simulink model "Simulatore_COMAU_UBHAND_IV_manipolazione_interna"  (Simulink 7.6)
settings: Solver=ode45  StartTime=0.0  StopTime=inf  RelTol=1e-3
values from neighbouring files (symbols this model uses):
  a_f = zeros(4,5)    [KinematicData.m]
  alpha_f = zeros(4,5)    [KinematicData.m]
  d_f = zeros(4,5)    [KinematicData.m]
  dc4_1 = 660    [KinematicData.m]
  T6HAND = [1 0 0 0;0 1 0 0; 0 0 1 357.48+95; 0 0 0 1]    [KinematicData.m]
  teta_d = atan(647.07/130)    [KinematicData.m]
  teta_f = zeros(4,5)    [KinematicData.m]
undefined symbols (not defined in the file or in its folder): a_c, a_w, alpha_c, alpha_w, d_c, d_w, Dt_fingers, teta_c
libraries: simulink x281, dspmtrx3 x171, aerolibutil x163, aerolibconvert2 x64, simulink_extras x46, vrlib x26, aerolibtransform2 x7, dspnetwork x1
(part 15 of 15)

== system Simulatore_COMAU_UBHAND_IV_manipolazione_interna/Work Space Control/Inverse_kinematic/Inverse_kinematic_comau/R_0index/T_6_index_^-1/Direct_kinematic_index1/Subsystem3 ==
  q : Inport
  alpha : Inport  Port=2
  a : Inport  Port=3
  A_01 : aerolibutil/Create 3x3 Matrix
  Constant : Constant  Value=0
  Constant1 : Constant  Value=[0 0 0 1]  VectorParams1D=off
  Constant5 : Constant  Value=0
  Display : Display
  Gain : Gain  Gain=-1
  Gain1 : Gain  Gain=-1
  Matrix Concatenation : Concatenate  Mode=Multidimensional array  ConcatenateDimension=2
  Matrix Concatenation1 : Concatenate  Mode=Multidimensional array
  Product{,1..5} (6 alike) : Product  RndMeth=Zero
  Trigonometric Function{,1,6..8,11..12} (7 alike) : Trigonometry  Operator=cos
  Trigonometric Function{2..5,9..10,13} (7 alike) : Trigonometry
  Vector Concatenate : Concatenate  NumInputs=3  Mode=Multidimensional array
  Out1 : Outport
  wires:
  Trigonometric Function2/1 -> Product/1
  Trigonometric Function1/1 -> Product/2
  q/1 -> Trigonometric Function/1, Trigonometric Function2/1, Trigonometric Function3/1, Trigonometric Function5/1, Trigonometric Function6/1, Trigonometric Function8/1, Trigonometric Function12/1, Trigonometric Function13/1
  alpha/1 -> Trigonometric Function1/1, Trigonometric Function4/1, Trigonometric Function7/1, Trigonometric Function9/1, Trigonometric Function10/1, Trigonometric Function11/1
  Trigonometric Function3/1 -> Product1/1
  Trigonometric Function4/1 -> Product1/2
  Trigonometric Function6/1 -> Product2/1
  Trigonometric Function7/1 -> Product2/2
  Trigonometric Function8/1 -> Product3/1
  Trigonometric Function9/1 -> Product3/2
  Product3/1 -> Gain1/1
  Product/1 -> Gain/1
  Trigonometric Function/1 -> A_01/1
  Gain/1 -> A_01/2
  Product1/1 -> A_01/3
  Trigonometric Function5/1 -> A_01/4
  Product2/1 -> A_01/5
  Gain1/1 -> A_01/6
  Constant/1 -> A_01/7
  Trigonometric Function10/1 -> A_01/8
  Trigonometric Function11/1 -> A_01/9
  a/1 -> Product5/1, Product4/1
  Trigonometric Function12/1 -> Product4/2
  Trigonometric Function13/1 -> Product5/2
  Product4/1 -> Vector Concatenate/1
  Product5/1 -> Vector Concatenate/2
  Constant5/1 -> Vector Concatenate/3
  A_01/1 -> Matrix Concatenation/1
  Vector Concatenate/1 -> Matrix Concatenation/2
  Matrix Concatenation/1 -> Matrix Concatenation1/1
  Constant1/1 -> Matrix Concatenation1/2
  Matrix Concatenation1/1 -> Out1/1, Display/1

== system Simulatore_COMAU_UBHAND_IV_manipolazione_interna/Work Space Control/Inverse_kinematic/Inverse_kinematic_comau/R_0index/T_6_index_^-1/Direct_kinematic_wrist1/T_6_8 ==
  wrist_angles[1..2] : Inport
  T_6H : Inport  Port=2
  Add : Sum
  Add1 : Sum
  Constant : Constant  Value=[alpha_w(1) alpha_w(2)]
  Constant1 : Constant  Value=0
  Constant2 : Constant  Value=[a_w(1) a_w(2)]
  Constant3 : Constant  Value=0
  Degrees to Radians : simulink_extras/Transformations/Degrees to Radians
  Demux : Demux  Outputs=2
  Demux1 : Demux  Outputs=2
  Demux2 : Demux  Outputs=2
  Display3 : Display
  Matrix Multiply : Product  Multiplication=Matrix(*)
  Matrix Multiply1 : Product  Multiplication=Matrix(*)
  Matrix Multiply2 : Product  Multiplication=Matrix(*)
  Subsystem : Subsystem
  Subsystem1 : Subsystem
  wrist_reference_ : Subsystem
  T_68 : Outport
  wires:
  wrist_angles[1..2]/1 -> Display3/1, Degrees to Radians/1
  Degrees to Radians/1 -> Demux/1
  Demux1/2 -> Subsystem1/2(alpha)
  Demux1/1 -> Subsystem/2(alpha)
  Demux2/2 -> Subsystem1/3(a)
  Demux2/1 -> Subsystem/3(a)
  Constant2/1 -> Demux2/1
  Matrix Multiply/1 -> T_68/1
  Subsystem1/1(Out1) -> Matrix Multiply/2
  Constant/1 -> Demux1/1
  Subsystem/1(Out1) -> Matrix Multiply1/2
  Matrix Multiply1/1 -> Matrix Multiply/1
  Constant1/1 -> Add/2
  Demux/1 -> Add/1
  Add/1 -> Subsystem/1(q)
  Constant3/1 -> Add1/2
  Demux/2 -> Add1/1
  Add1/1 -> Subsystem1/1(q)
  T_6H/1 -> Matrix Multiply2/1
  wrist_reference_/1(T_HW) -> Matrix Multiply2/2
  Matrix Multiply2/1 -> Matrix Multiply1/1

== system Simulatore_COMAU_UBHAND_IV_manipolazione_interna/Work Space Control/Inverse_kinematic/Inverse_kinematic_comau/R_0index/T_6_object^-1/Direct_kinematic_index1/Subsystem ==
  q : Inport
  alpha : Inport  Port=2
  a : Inport  Port=3
  A_01 : aerolibutil/Create 3x3 Matrix
  Constant : Constant  Value=0
  Constant1 : Constant  Value=[0 0 0 1]  VectorParams1D=off
  Constant5 : Constant  Value=0
  Display : Display
  Gain : Gain  Gain=-1
  Gain1 : Gain  Gain=-1
  Matrix Concatenation : Concatenate  Mode=Multidimensional array  ConcatenateDimension=2
  Matrix Concatenation1 : Concatenate  Mode=Multidimensional array
  Product{,1..5} (6 alike) : Product  RndMeth=Zero
  Trigonometric Function{,1,6..8,11..12} (7 alike) : Trigonometry  Operator=cos
  Trigonometric Function{2..5,9..10,13} (7 alike) : Trigonometry
  Vector Concatenate : Concatenate  NumInputs=3  Mode=Multidimensional array
  Out1 : Outport
  wires:
  Trigonometric Function2/1 -> Product/1
  Trigonometric Function1/1 -> Product/2
  q/1 -> Trigonometric Function/1, Trigonometric Function2/1, Trigonometric Function3/1, Trigonometric Function5/1, Trigonometric Function6/1, Trigonometric Function8/1, Trigonometric Function12/1, Trigonometric Function13/1
  alpha/1 -> Trigonometric Function1/1, Trigonometric Function4/1, Trigonometric Function7/1, Trigonometric Function9/1, Trigonometric Function10/1, Trigonometric Function11/1
  Trigonometric Function3/1 -> Product1/1
  Trigonometric Function4/1 -> Product1/2
  Trigonometric Function6/1 -> Product2/1
  Trigonometric Function7/1 -> Product2/2
  Trigonometric Function8/1 -> Product3/1
  Trigonometric Function9/1 -> Product3/2
  Product3/1 -> Gain1/1
  Product/1 -> Gain/1
  Trigonometric Function/1 -> A_01/1
  Gain/1 -> A_01/2
  Product1/1 -> A_01/3
  Trigonometric Function5/1 -> A_01/4
  Product2/1 -> A_01/5
  Gain1/1 -> A_01/6
  Constant/1 -> A_01/7
  Trigonometric Function10/1 -> A_01/8
  Trigonometric Function11/1 -> A_01/9
  a/1 -> Product4/1, Product5/1
  Trigonometric Function12/1 -> Product4/2
  Trigonometric Function13/1 -> Product5/2
  Product4/1 -> Vector Concatenate/1
  Product5/1 -> Vector Concatenate/2
  Constant5/1 -> Vector Concatenate/3
  A_01/1 -> Matrix Concatenation/1
  Vector Concatenate/1 -> Matrix Concatenation/2
  Matrix Concatenation/1 -> Matrix Concatenation1/1
  Constant1/1 -> Matrix Concatenation1/2
  Matrix Concatenation1/1 -> Out1/1, Display/1

== system Simulatore_COMAU_UBHAND_IV_manipolazione_interna/Work Space Control/Inverse_kinematic/Inverse_kinematic_comau/R_0index/T_6_object^-1/Direct_kinematic_index1/Subsystem1 ==
  q : Inport
  alpha : Inport  Port=2
  a : Inport  Port=3
  A_01 : aerolibutil/Create 3x3 Matrix
  Constant : Constant  Value=0
  Constant1 : Constant  Value=[0 0 0 1]  VectorParams1D=off
  Constant5 : Constant  Value=0
  Display : Display
  Gain : Gain  Gain=-1
  Gain1 : Gain  Gain=-1
  Matrix Concatenation : Concatenate  Mode=Multidimensional array  ConcatenateDimension=2
  Matrix Concatenation1 : Concatenate  Mode=Multidimensional array
  Product{,1..5} (6 alike) : Product  RndMeth=Zero
  Trigonometric Function{,1,6..8,11..12} (7 alike) : Trigonometry  Operator=cos
  Trigonometric Function{2..5,9..10,13} (7 alike) : Trigonometry
  Vector Concatenate : Concatenate  NumInputs=3  Mode=Multidimensional array
  Out1 : Outport
  wires:
  Matrix Concatenation1/1 -> Display/1, Out1/1
  Constant1/1 -> Matrix Concatenation1/2
  Matrix Concatenation/1 -> Matrix Concatenation1/1
  Vector Concatenate/1 -> Matrix Concatenation/2
  A_01/1 -> Matrix Concatenation/1
  Constant5/1 -> Vector Concatenate/3
  Product5/1 -> Vector Concatenate/2
  Product4/1 -> Vector Concatenate/1
  Trigonometric Function13/1 -> Product5/2
  Trigonometric Function12/1 -> Product4/2
  a/1 -> Product4/1, Product5/1
  Trigonometric Function11/1 -> A_01/9
  Trigonometric Function10/1 -> A_01/8
  Constant/1 -> A_01/7
  Gain1/1 -> A_01/6
  Product2/1 -> A_01/5
  Trigonometric Function5/1 -> A_01/4
  Product1/1 -> A_01/3
  Gain/1 -> A_01/2
  Trigonometric Function/1 -> A_01/1
  Product/1 -> Gain/1
  Product3/1 -> Gain1/1
  Trigonometric Function9/1 -> Product3/2
  Trigonometric Function8/1 -> Product3/1
  Trigonometric Function7/1 -> Product2/2
  Trigonometric Function6/1 -> Product2/1
  Trigonometric Function4/1 -> Product1/2
  Trigonometric Function3/1 -> Product1/1
  alpha/1 -> Trigonometric Function11/1, Trigonometric Function10/1, Trigonometric Function9/1, Trigonometric Function7/1, Trigonometric Function4/1, Trigonometric Function1/1
  q/1 -> Trigonometric Function13/1, Trigonometric Function12/1, Trigonometric Function8/1, Trigonometric Function6/1, Trigonometric Function5/1, Trigonometric Function3/1, Trigonometric Function2/1, Trigonometric Function/1
  Trigonometric Function1/1 -> Product/2
  Trigonometric Function2/1 -> Product/1

== system Simulatore_COMAU_UBHAND_IV_manipolazione_interna/Work Space Control/Inverse_kinematic/Inverse_kinematic_comau/R_0index/T_6_object^-1/Direct_kinematic_index1/Subsystem2 ==
  q : Inport
  alpha : Inport  Port=2
  a : Inport  Port=3
  A_01 : aerolibutil/Create 3x3 Matrix
  Constant : Constant  Value=0
  Constant1 : Constant  Value=[0 0 0 1]  VectorParams1D=off
  Constant5 : Constant  Value=0
  Display : Display
  Gain : Gain  Gain=-1
  Gain1 : Gain  Gain=-1
  Matrix Concatenation : Concatenate  Mode=Multidimensional array  ConcatenateDimension=2
  Matrix Concatenation1 : Concatenate  Mode=Multidimensional array
  Product{,1..5} (6 alike) : Product  RndMeth=Zero
  Trigonometric Function{,1,6..8,11..12} (7 alike) : Trigonometry  Operator=cos
  Trigonometric Function{2..5,9..10,13} (7 alike) : Trigonometry
  Vector Concatenate : Concatenate  NumInputs=3  Mode=Multidimensional array
  Out1 : Outport
  wires:
  Trigonometric Function2/1 -> Product/1
  Trigonometric Function1/1 -> Product/2
  q/1 -> Trigonometric Function/1, Trigonometric Function2/1, Trigonometric Function3/1, Trigonometric Function5/1, Trigonometric Function6/1, Trigonometric Function8/1, Trigonometric Function12/1, Trigonometric Function13/1
  alpha/1 -> Trigonometric Function1/1, Trigonometric Function4/1, Trigonometric Function7/1, Trigonometric Function9/1, Trigonometric Function10/1, Trigonometric Function11/1
  Trigonometric Function3/1 -> Product1/1
  Trigonometric Function4/1 -> Product1/2
  Trigonometric Function6/1 -> Product2/1
  Trigonometric Function7/1 -> Product2/2
  Trigonometric Function8/1 -> Product3/1
  Trigonometric Function9/1 -> Product3/2
  Product3/1 -> Gain1/1
  Product/1 -> Gain/1
  Trigonometric Function/1 -> A_01/1
  Gain/1 -> A_01/2
  Product1/1 -> A_01/3
  Trigonometric Function5/1 -> A_01/4
  Product2/1 -> A_01/5
  Gain1/1 -> A_01/6
  Constant/1 -> A_01/7
  Trigonometric Function10/1 -> A_01/8
  Trigonometric Function11/1 -> A_01/9
  a/1 -> Product4/1, Product5/1
  Trigonometric Function12/1 -> Product4/2
  Trigonometric Function13/1 -> Product5/2
  Product4/1 -> Vector Concatenate/1
  Product5/1 -> Vector Concatenate/2
  Constant5/1 -> Vector Concatenate/3
  A_01/1 -> Matrix Concatenation/1
  Vector Concatenate/1 -> Matrix Concatenation/2
  Matrix Concatenation/1 -> Matrix Concatenation1/1
  Constant1/1 -> Matrix Concatenation1/2
  Matrix Concatenation1/1 -> Out1/1, Display/1

== system Simulatore_COMAU_UBHAND_IV_manipolazione_interna/Work Space Control/Inverse_kinematic/Inverse_kinematic_comau/R_0index/T_6_object^-1/Direct_kinematic_index1/Subsystem3 ==
  q : Inport
  alpha : Inport  Port=2
  a : Inport  Port=3
  A_01 : aerolibutil/Create 3x3 Matrix
  Constant : Constant  Value=0
  Constant1 : Constant  Value=[0 0 0 1]  VectorParams1D=off
  Constant5 : Constant  Value=0
  Display : Display
  Gain : Gain  Gain=-1
  Gain1 : Gain  Gain=-1
  Matrix Concatenation : Concatenate  Mode=Multidimensional array  ConcatenateDimension=2
  Matrix Concatenation1 : Concatenate  Mode=Multidimensional array
  Product{,1..5} (6 alike) : Product  RndMeth=Zero
  Trigonometric Function{,1,6..8,11..12} (7 alike) : Trigonometry  Operator=cos
  Trigonometric Function{2..5,9..10,13} (7 alike) : Trigonometry
  Vector Concatenate : Concatenate  NumInputs=3  Mode=Multidimensional array
  Out1 : Outport
  wires:
  Matrix Concatenation1/1 -> Display/1, Out1/1
  Constant1/1 -> Matrix Concatenation1/2
  Matrix Concatenation/1 -> Matrix Concatenation1/1
  Vector Concatenate/1 -> Matrix Concatenation/2
  A_01/1 -> Matrix Concatenation/1
  Constant5/1 -> Vector Concatenate/3
  Product5/1 -> Vector Concatenate/2
  Product4/1 -> Vector Concatenate/1
  Trigonometric Function13/1 -> Product5/2
  Trigonometric Function12/1 -> Product4/2
  a/1 -> Product4/1, Product5/1
  Trigonometric Function11/1 -> A_01/9
  Trigonometric Function10/1 -> A_01/8
  Constant/1 -> A_01/7
  Gain1/1 -> A_01/6
  Product2/1 -> A_01/5
  Trigonometric Function5/1 -> A_01/4
  Product1/1 -> A_01/3
  Gain/1 -> A_01/2
  Trigonometric Function/1 -> A_01/1
  Product/1 -> Gain/1
  Product3/1 -> Gain1/1
  Trigonometric Function9/1 -> Product3/2
  Trigonometric Function8/1 -> Product3/1
  Trigonometric Function7/1 -> Product2/2
  Trigonometric Function6/1 -> Product2/1
  Trigonometric Function4/1 -> Product1/2
  Trigonometric Function3/1 -> Product1/1
  alpha/1 -> Trigonometric Function11/1, Trigonometric Function10/1, Trigonometric Function9/1, Trigonometric Function7/1, Trigonometric Function4/1, Trigonometric Function1/1
  q/1 -> Trigonometric Function13/1, Trigonometric Function12/1, Trigonometric Function8/1, Trigonometric Function6/1, Trigonometric Function5/1, Trigonometric Function3/1, Trigonometric Function2/1, Trigonometric Function/1
  Trigonometric Function1/1 -> Product/2
  Trigonometric Function2/1 -> Product/1

== system Simulatore_COMAU_UBHAND_IV_manipolazione_interna/Work Space Control/Inverse_kinematic/Inverse_kinematic_comau/R_0index/T_6_object^-1/Direct_kinematic_wrist1/T_6_8 ==
  wrist_angles[1..2] : Inport
  T_6H : Inport  Port=2
  Add : Sum
  Add1 : Sum
  Constant : Constant  Value=[alpha_w(1) alpha_w(2)]
  Constant1 : Constant  Value=0
  Constant2 : Constant  Value=[a_w(1) a_w(2)]
  Constant3 : Constant  Value=0
  Degrees to Radians : simulink_extras/Transformations/Degrees to Radians
  Demux : Demux  Outputs=2
  Demux1 : Demux  Outputs=2
  Demux2 : Demux  Outputs=2
  Display3 : Display
  Matrix Multiply : Product  Multiplication=Matrix(*)
  Matrix Multiply1 : Product  Multiplication=Matrix(*)
  Matrix Multiply2 : Product  Multiplication=Matrix(*)
  Subsystem : Subsystem
  Subsystem1 : Subsystem
  wrist_reference_ : Subsystem
  T_68 : Outport
  wires:
  Matrix Multiply2/1 -> Matrix Multiply1/1
  wrist_reference_/1(T_HW) -> Matrix Multiply2/2
  T_6H/1 -> Matrix Multiply2/1
  Add1/1 -> Subsystem1/1(q)
  Demux/2 -> Add1/1
  Constant3/1 -> Add1/2
  Add/1 -> Subsystem/1(q)
  Demux/1 -> Add/1
  Constant1/1 -> Add/2
  Matrix Multiply1/1 -> Matrix Multiply/1
  Subsystem/1(Out1) -> Matrix Multiply1/2
  Constant/1 -> Demux1/1
  Subsystem1/1(Out1) -> Matrix Multiply/2
  Matrix Multiply/1 -> T_68/1
  Constant2/1 -> Demux2/1
  Demux2/1 -> Subsystem/3(a)
  Demux2/2 -> Subsystem1/3(a)
  Demux1/1 -> Subsystem/2(alpha)
  Demux1/2 -> Subsystem1/2(alpha)
  Degrees to Radians/1 -> Demux/1
  wrist_angles[1..2]/1 -> Degrees to Radians/1, Display3/1

== system Simulatore_COMAU_UBHAND_IV_manipolazione_interna/Work Space Control/Inverse_kinematic/Inverse_kinematic_hand/Inverse_kinematic_little/jacobian/z1/pe-P(i-i) ==
  T_03 : Inport
  T_01 : Inport  Port=2
  T_02 : Inport  Port=3
  T_base : Inport  Port=4
  Add : Sum  Inputs=+-
  Add1 : Sum  Inputs=+-
  Add2 : Sum  Inputs=+-
  R36(1,3){1,6..8} (4 alike) : dspmtrx3/Submatrix  RowSpan=Range of rows  RowStartMode=Index  RowStartIndex=1  RowEndMode=Index  RowEndIndex=3  ColSpan=One column  ColStartMode=Index  ColStartIndex=4  ColEndMode=Offset from last  ColEndIndex=1
  pe-p0 : Outport
  pe-p1 : Outport  Port=2
  pe-p2 : Outport  Port=3
  wires:
  T_base/1 -> R36(1,3)1/1
  Add2/1 -> pe-p2/1
  Add1/1 -> pe-p1/1
  Add/1 -> pe-p0/1
  R36(1,3)8/1 -> Add2/2
  R36(1,3)7/1 -> Add1/2
  T_02/1 -> R36(1,3)8/1
  T_01/1 -> R36(1,3)7/1
  R36(1,3)1/1 -> Add/2
  R36(1,3)6/1 -> Add1/1, Add2/1, Add/1
  T_03/1 -> R36(1,3)6/1

== system Simulatore_COMAU_UBHAND_IV_manipolazione_interna/Work Space Control/Inverse_kinematic/Inverse_kinematic_hand/Inverse_kinematic_little/jacobian/z1/z(i-1) ==
  T_01 : Inport
  T_02 : Inport  Port=2
  T_base : Inport  Port=3
  R36(1,3) : dspmtrx3/Submatrix  RowSpan=Range of rows  RowStartMode=Index  RowStartIndex=1  RowEndMode=Index  RowEndIndex=3  ColSpan=One column  ColStartMode=Index  ColStartIndex=3  ColEndMode=Offset from last  ColEndIndex=1
  R36(1,3)1 : dspmtrx3/Submatrix  RowSpan=Range of rows  RowStartMode=Index  RowStartIndex=1  RowEndMode=Index  RowEndIndex=3  ColSpan=One column  ColStartMode=Index  ColStartIndex=3  ColEndMode=Offset from last  ColEndIndex=1
  R36(1,3)5 : dspmtrx3/Submatrix  RowSpan=Range of rows  RowStartMode=Index  RowStartIndex=1  RowEndMode=Index  RowEndIndex=3  ColSpan=One column  ColStartMode=Index  ColStartIndex=3  ColEndMode=Offset from last  ColEndIndex=1
  z0 : Outport
  z1 : Outport  Port=2
  z2 : Outport  Port=3
  wires:
  R36(1,3)5/1 -> z0/1
  T_base/1 -> R36(1,3)5/1
  R36(1,3)1/1 -> z2/1
  R36(1,3)/1 -> z1/1
  T_01/1 -> R36(1,3)/1
  T_02/1 -> R36(1,3)1/1

== system Simulatore_COMAU_UBHAND_IV_manipolazione_interna/Work Space Control/Inverse_kinematic/Inverse_kinematic_hand/Inverse_kinematic_middle/jacobian/z1/pe-P(i-i) ==
  T_03 : Inport
  T_01 : Inport  Port=2
  T_02 : Inport  Port=3
  T_base : Inport  Port=4
  Add : Sum  Inputs=+-
  Add1 : Sum  Inputs=+-
  Add2 : Sum  Inputs=+-
  R36(1,3){1,6..8} (4 alike) : dspmtrx3/Submatrix  RowSpan=Range of rows  RowStartMode=Index  RowStartIndex=1  RowEndMode=Index  RowEndIndex=3  ColSpan=One column  ColStartMode=Index  ColStartIndex=4  ColEndMode=Offset from last  ColEndIndex=1
  pe-p0 : Outport
  pe-p1 : Outport  Port=2
  pe-p2 : Outport  Port=3
  wires:
  T_base/1 -> R36(1,3)1/1
  Add2/1 -> pe-p2/1
  Add1/1 -> pe-p1/1
  Add/1 -> pe-p0/1
  R36(1,3)8/1 -> Add2/2
  R36(1,3)7/1 -> Add1/2
  T_02/1 -> R36(1,3)8/1
  T_01/1 -> R36(1,3)7/1
  R36(1,3)1/1 -> Add/2
  R36(1,3)6/1 -> Add1/1, Add2/1, Add/1
  T_03/1 -> R36(1,3)6/1

== system Simulatore_COMAU_UBHAND_IV_manipolazione_interna/Work Space Control/Inverse_kinematic/Inverse_kinematic_hand/Inverse_kinematic_middle/jacobian/z1/z(i-1) ==
  T_01 : Inport
  T_02 : Inport  Port=2
  T_base : Inport  Port=3
  R36(1,3) : dspmtrx3/Submatrix  RowSpan=Range of rows  RowStartMode=Index  RowStartIndex=1  RowEndMode=Index  RowEndIndex=3  ColSpan=One column  ColStartMode=Index  ColStartIndex=3  ColEndMode=Offset from last  ColEndIndex=1
  R36(1,3)1 : dspmtrx3/Submatrix  RowSpan=Range of rows  RowStartMode=Index  RowStartIndex=1  RowEndMode=Index  RowEndIndex=3  ColSpan=One column  ColStartMode=Index  ColStartIndex=3  ColEndMode=Offset from last  ColEndIndex=1
  R36(1,3)5 : dspmtrx3/Submatrix  RowSpan=Range of rows  RowStartMode=Index  RowStartIndex=1  RowEndMode=Index  RowEndIndex=3  ColSpan=One column  ColStartMode=Index  ColStartIndex=3  ColEndMode=Offset from last  ColEndIndex=1
  z0 : Outport
  z1 : Outport  Port=2
  z2 : Outport  Port=3
  wires:
  R36(1,3)5/1 -> z0/1
  T_base/1 -> R36(1,3)5/1
  R36(1,3)1/1 -> z2/1
  R36(1,3)/1 -> z1/1
  T_01/1 -> R36(1,3)/1
  T_02/1 -> R36(1,3)1/1

== system Simulatore_COMAU_UBHAND_IV_manipolazione_interna/Work Space Control/Inverse_kinematic/Inverse_kinematic_hand/Inverse_kinematic_middle1/jacobian/z1/pe-P(i-i) ==
  T_03 : Inport
  T_01 : Inport  Port=2
  T_02 : Inport  Port=3
  T_base : Inport  Port=4
  Add : Sum  Inputs=+-
  Add1 : Sum  Inputs=+-
  Add2 : Sum  Inputs=+-
  R36(1,3){1,6..8} (4 alike) : dspmtrx3/Submatrix  RowSpan=Range of rows  RowStartMode=Index  RowStartIndex=1  RowEndMode=Index  RowEndIndex=3  ColSpan=One column  ColStartMode=Index  ColStartIndex=4  ColEndMode=Offset from last  ColEndIndex=1
  pe-p0 : Outport
  pe-p1 : Outport  Port=2
  pe-p2 : Outport  Port=3
  wires:
  T_base/1 -> R36(1,3)1/1
  Add2/1 -> pe-p2/1
  Add1/1 -> pe-p1/1
  Add/1 -> pe-p0/1
  R36(1,3)8/1 -> Add2/2
  R36(1,3)7/1 -> Add1/2
  T_02/1 -> R36(1,3)8/1
  T_01/1 -> R36(1,3)7/1
  R36(1,3)1/1 -> Add/2
  R36(1,3)6/1 -> Add1/1, Add2/1, Add/1
  T_03/1 -> R36(1,3)6/1

== system Simulatore_COMAU_UBHAND_IV_manipolazione_interna/Work Space Control/Inverse_kinematic/Inverse_kinematic_hand/Inverse_kinematic_middle1/jacobian/z1/z(i-1) ==
  T_01 : Inport
  T_02 : Inport  Port=2
  T_base : Inport  Port=3
  R36(1,3) : dspmtrx3/Submatrix  RowSpan=Range of rows  RowStartMode=Index  RowStartIndex=1  RowEndMode=Index  RowEndIndex=3  ColSpan=One column  ColStartMode=Index  ColStartIndex=3  ColEndMode=Offset from last  ColEndIndex=1
  R36(1,3)1 : dspmtrx3/Submatrix  RowSpan=Range of rows  RowStartMode=Index  RowStartIndex=1  RowEndMode=Index  RowEndIndex=3  ColSpan=One column  ColStartMode=Index  ColStartIndex=3  ColEndMode=Offset from last  ColEndIndex=1
  R36(1,3)5 : dspmtrx3/Submatrix  RowSpan=Range of rows  RowStartMode=Index  RowStartIndex=1  RowEndMode=Index  RowEndIndex=3  ColSpan=One column  ColStartMode=Index  ColStartIndex=3  ColEndMode=Offset from last  ColEndIndex=1
  z0 : Outport
  z1 : Outport  Port=2
  z2 : Outport  Port=3
  wires:
  R36(1,3)5/1 -> z0/1
  T_base/1 -> R36(1,3)5/1
  R36(1,3)1/1 -> z2/1
  R36(1,3)/1 -> z1/1
  T_01/1 -> R36(1,3)/1
  T_02/1 -> R36(1,3)1/1

== system Simulatore_COMAU_UBHAND_IV_manipolazione_interna/Work Space Control/Inverse_kinematic/Inverse_kinematic_hand/Inverse_kinematic_ring/jacobian/z1/pe-P(i-i) ==
  T_03 : Inport
  T_01 : Inport  Port=2
  T_02 : Inport  Port=3
  T_base : Inport  Port=4
  Add : Sum  Inputs=+-
  Add1 : Sum  Inputs=+-
  Add2 : Sum  Inputs=+-
  R36(1,3){1,6..8} (4 alike) : dspmtrx3/Submatrix  RowSpan=Range of rows  RowStartMode=Index  RowStartIndex=1  RowEndMode=Index  RowEndIndex=3  ColSpan=One column  ColStartMode=Index  ColStartIndex=4  ColEndMode=Offset from last  ColEndIndex=1
  pe-p0 : Outport
  pe-p1 : Outport  Port=2
  pe-p2 : Outport  Port=3
  wires:
  T_base/1 -> R36(1,3)1/1
  Add2/1 -> pe-p2/1
  Add1/1 -> pe-p1/1
  Add/1 -> pe-p0/1
  R36(1,3)8/1 -> Add2/2
  R36(1,3)7/1 -> Add1/2
  T_02/1 -> R36(1,3)8/1
  T_01/1 -> R36(1,3)7/1
  R36(1,3)1/1 -> Add/2
  R36(1,3)6/1 -> Add1/1, Add2/1, Add/1
  T_03/1 -> R36(1,3)6/1

== system Simulatore_COMAU_UBHAND_IV_manipolazione_interna/Work Space Control/Inverse_kinematic/Inverse_kinematic_hand/Inverse_kinematic_ring/jacobian/z1/z(i-1) ==
  T_01 : Inport
  T_02 : Inport  Port=2
  T_base : Inport  Port=3
  R36(1,3) : dspmtrx3/Submatrix  RowSpan=Range of rows  RowStartMode=Index  RowStartIndex=1  RowEndMode=Index  RowEndIndex=3  ColSpan=One column  ColStartMode=Index  ColStartIndex=3  ColEndMode=Offset from last  ColEndIndex=1
  R36(1,3)1 : dspmtrx3/Submatrix  RowSpan=Range of rows  RowStartMode=Index  RowStartIndex=1  RowEndMode=Index  RowEndIndex=3  ColSpan=One column  ColStartMode=Index  ColStartIndex=3  ColEndMode=Offset from last  ColEndIndex=1
  R36(1,3)5 : dspmtrx3/Submatrix  RowSpan=Range of rows  RowStartMode=Index  RowStartIndex=1  RowEndMode=Index  RowEndIndex=3  ColSpan=One column  ColStartMode=Index  ColStartIndex=3  ColEndMode=Offset from last  ColEndIndex=1
  z0 : Outport
  z1 : Outport  Port=2
  z2 : Outport  Port=3
  wires:
  R36(1,3)5/1 -> z0/1
  T_base/1 -> R36(1,3)5/1
  R36(1,3)1/1 -> z2/1
  R36(1,3)/1 -> z1/1
  T_01/1 -> R36(1,3)/1
  T_02/1 -> R36(1,3)1/1

== system Simulatore_COMAU_UBHAND_IV_manipolazione_interna/Work Space Control/Inverse_kinematic/Inverse_kinematic_hand/Inverse_kinematic_thumb/jacobian/z1/pe-P(i-i) ==
  T_03 : Inport
  T_01 : Inport  Port=2
  T_02 : Inport  Port=3
  T_base : Inport  Port=4
  Add : Sum  Inputs=+-
  Add1 : Sum  Inputs=+-
  Add2 : Sum  Inputs=+-
  R36(1,3){1,6..8} (4 alike) : dspmtrx3/Submatrix  RowSpan=Range of rows  RowStartMode=Index  RowStartIndex=1  RowEndMode=Index  RowEndIndex=3  ColSpan=One column  ColStartMode=Index  ColStartIndex=4  ColEndMode=Offset from last  ColEndIndex=1
  pe-p0 : Outport
  pe-p1 : Outport  Port=2
  pe-p2 : Outport  Port=3
  wires:
  T_base/1 -> R36(1,3)1/1
  Add2/1 -> pe-p2/1
  Add1/1 -> pe-p1/1
  Add/1 -> pe-p0/1
  R36(1,3)8/1 -> Add2/2
  R36(1,3)7/1 -> Add1/2
  T_02/1 -> R36(1,3)8/1
  T_01/1 -> R36(1,3)7/1
  R36(1,3)1/1 -> Add/2
  R36(1,3)6/1 -> Add1/1, Add2/1, Add/1
  T_03/1 -> R36(1,3)6/1

== system Simulatore_COMAU_UBHAND_IV_manipolazione_interna/Work Space Control/Inverse_kinematic/Inverse_kinematic_hand/Inverse_kinematic_thumb/jacobian/z1/z(i-1) ==
  T_01 : Inport
  T_02 : Inport  Port=2
  T_base : Inport  Port=3
  R36(1,3) : dspmtrx3/Submatrix  RowSpan=Range of rows  RowStartMode=Index  RowStartIndex=1  RowEndMode=Index  RowEndIndex=3  ColSpan=One column  ColStartMode=Index  ColStartIndex=3  ColEndMode=Offset from last  ColEndIndex=1
  R36(1,3)1 : dspmtrx3/Submatrix  RowSpan=Range of rows  RowStartMode=Index  RowStartIndex=1  RowEndMode=Index  RowEndIndex=3  ColSpan=One column  ColStartMode=Index  ColStartIndex=3  ColEndMode=Offset from last  ColEndIndex=1
  R36(1,3)5 : dspmtrx3/Submatrix  RowSpan=Range of rows  RowStartMode=Index  RowStartIndex=1  RowEndMode=Index  RowEndIndex=3  ColSpan=One column  ColStartMode=Index  ColStartIndex=3  ColEndMode=Offset from last  ColEndIndex=1
  z0 : Outport
  z1 : Outport  Port=2
  z2 : Outport  Port=3
  wires:
  R36(1,3)5/1 -> z0/1
  T_base/1 -> R36(1,3)5/1
  R36(1,3)1/1 -> z2/1
  R36(1,3)/1 -> z1/1
  T_01/1 -> R36(1,3)/1
  T_02/1 -> R36(1,3)1/1

== system Simulatore_COMAU_UBHAND_IV_manipolazione_interna/Work Space Control/Inverse_kinematic/Inverse_kinematic_comau/R_0index/T_0index/Direct_kinematic_wrist/T_6_8/Subsystem ==
  q : Inport
  alpha : Inport  Port=2
  a : Inport  Port=3
  A_01 : aerolibutil/Create 3x3 Matrix
  Constant : Constant  Value=0
  Constant1 : Constant  Value=[0 0 0 1]  VectorParams1D=off
  Constant5 : Constant  Value=d_w(1)
  Display : Display
  Gain : Gain  Gain=-1
  Gain1 : Gain  Gain=-1
  Matrix Concatenation : Concatenate  Mode=Multidimensional array  ConcatenateDimension=2
  Matrix Concatenation1 : Concatenate  Mode=Multidimensional array
  Product{,1..5} (6 alike) : Product  RndMeth=Zero
  Trigonometric Function{,1,6..8,11..12} (7 alike) : Trigonometry  Operator=cos
  Trigonometric Function{2..5,9..10,13} (7 alike) : Trigonometry
  Vector Concatenate : Concatenate  NumInputs=3  Mode=Multidimensional array
  Out1 : Outport
  wires:
  Matrix Concatenation1/1 -> Display/1, Out1/1
  Constant1/1 -> Matrix Concatenation1/2
  Matrix Concatenation/1 -> Matrix Concatenation1/1
  Vector Concatenate/1 -> Matrix Concatenation/2
  A_01/1 -> Matrix Concatenation/1
  Constant5/1 -> Vector Concatenate/3
  Product5/1 -> Vector Concatenate/2
  Product4/1 -> Vector Concatenate/1
  Trigonometric Function13/1 -> Product5/2
  Trigonometric Function12/1 -> Product4/2
  a/1 -> Product5/1, Product4/1
  Trigonometric Function11/1 -> A_01/9
  Trigonometric Function10/1 -> A_01/8
  Constant/1 -> A_01/7
  Gain1/1 -> A_01/6
  Product2/1 -> A_01/5
  Trigonometric Function5/1 -> A_01/4
  Product1/1 -> A_01/3
  Gain/1 -> A_01/2
  Trigonometric Function/1 -> A_01/1
  Product/1 -> Gain/1
  Product3/1 -> Gain1/1
  Trigonometric Function9/1 -> Product3/2
  Trigonometric Function8/1 -> Product3/1
  Trigonometric Function7/1 -> Product2/2
  Trigonometric Function6/1 -> Product2/1
  Trigonometric Function4/1 -> Product1/2
  Trigonometric Function3/1 -> Product1/1
  alpha/1 -> Trigonometric Function11/1, Trigonometric Function10/1, Trigonometric Function9/1, Trigonometric Function7/1, Trigonometric Function4/1, Trigonometric Function1/1
  q/1 -> Trigonometric Function13/1, Trigonometric Function12/1, Trigonometric Function8/1, Trigonometric Function6/1, Trigonometric Function5/1, Trigonometric Function3/1, Trigonometric Function2/1, Trigonometric Function/1
  Trigonometric Function1/1 -> Product/2
  Trigonometric Function2/1 -> Product/1

== system Simulatore_COMAU_UBHAND_IV_manipolazione_interna/Work Space Control/Inverse_kinematic/Inverse_kinematic_comau/R_0index/T_0index/Direct_kinematic_wrist/T_6_8/Subsystem1 ==
  q : Inport
  alpha : Inport  Port=2
  a : Inport  Port=3
  A_01 : aerolibutil/Create 3x3 Matrix
  Constant : Constant  Value=0
  Constant1 : Constant  Value=[0 0 0 1]  VectorParams1D=off
  Constant5 : Constant  Value=d_w(2)
  Display : Display
  Gain : Gain  Gain=-1
  Gain1 : Gain  Gain=-1
  Matrix Concatenation : Concatenate  Mode=Multidimensional array  ConcatenateDimension=2
  Matrix Concatenation1 : Concatenate  Mode=Multidimensional array
  Product{,1..5} (6 alike) : Product  RndMeth=Zero
  Trigonometric Function{,1,6..8,11..12} (7 alike) : Trigonometry  Operator=cos
  Trigonometric Function{2..5,9..10,13} (7 alike) : Trigonometry
  Vector Concatenate : Concatenate  NumInputs=3  Mode=Multidimensional array
  Out1 : Outport
  wires:
  Trigonometric Function2/1 -> Product/1
  Trigonometric Function1/1 -> Product/2
  q/1 -> Trigonometric Function/1, Trigonometric Function2/1, Trigonometric Function3/1, Trigonometric Function5/1, Trigonometric Function6/1, Trigonometric Function8/1, Trigonometric Function12/1, Trigonometric Function13/1
  alpha/1 -> Trigonometric Function1/1, Trigonometric Function4/1, Trigonometric Function7/1, Trigonometric Function9/1, Trigonometric Function10/1, Trigonometric Function11/1
  Trigonometric Function3/1 -> Product1/1
  Trigonometric Function4/1 -> Product1/2
  Trigonometric Function6/1 -> Product2/1
  Trigonometric Function7/1 -> Product2/2
  Trigonometric Function8/1 -> Product3/1
  Trigonometric Function9/1 -> Product3/2
  Product3/1 -> Gain1/1
  Product/1 -> Gain/1
  Trigonometric Function/1 -> A_01/1
  Gain/1 -> A_01/2
  Product1/1 -> A_01/3
  Trigonometric Function5/1 -> A_01/4
  Product2/1 -> A_01/5
  Gain1/1 -> A_01/6
  Constant/1 -> A_01/7
  Trigonometric Function10/1 -> A_01/8
  Trigonometric Function11/1 -> A_01/9
  a/1 -> Product5/1, Product4/1
  Trigonometric Function12/1 -> Product4/2
  Trigonometric Function13/1 -> Product5/2
  Product4/1 -> Vector Concatenate/1
  Product5/1 -> Vector Concatenate/2
  Constant5/1 -> Vector Concatenate/3
  A_01/1 -> Matrix Concatenation/1
  Vector Concatenate/1 -> Matrix Concatenation/2
  Matrix Concatenation/1 -> Matrix Concatenation1/1
  Constant1/1 -> Matrix Concatenation1/2
  Matrix Concatenation1/1 -> Out1/1, Display/1

== system Simulatore_COMAU_UBHAND_IV_manipolazione_interna/Work Space Control/Inverse_kinematic/Inverse_kinematic_comau/R_0index/T_0index/Direct_kinematic_wrist/T_6_8/wrist_reference_ ==
  Angle Conversion : aerolibconvert2/Angle Conversion  IU=deg  OU=rad
  Constant1 : Constant  Value=[0 90 0]  VectorParams1D=off
  Rotation Angles to Direction Cosine Matrix : aerolibtransform2/Rotation Angles to Direction Cosine Matrix  rotationOrder=ZYX
  R_HW : Outport
  wires:
  Rotation Angles to Direction Cosine Matrix/1 -> R_HW/1
  Angle Conversion/1 -> Rotation Angles to Direction Cosine Matrix/1
  Constant1/1 -> Angle Conversion/1

== system Simulatore_COMAU_UBHAND_IV_manipolazione_interna/Work Space Control/Inverse_kinematic/Inverse_kinematic_comau/R_0index/T_6_index_^-1/Direct_kinematic_wrist1/T_6_8/Subsystem ==
  q : Inport
  alpha : Inport  Port=2
  a : Inport  Port=3
  A_01 : aerolibutil/Create 3x3 Matrix
  Constant : Constant  Value=0
  Constant1 : Constant  Value=[0 0 0 1]  VectorParams1D=off
  Constant5 : Constant  Value=d_w(1)
  Display : Display
  Gain : Gain  Gain=-1
  Gain1 : Gain  Gain=-1
  Matrix Concatenation : Concatenate  Mode=Multidimensional array  ConcatenateDimension=2
  Matrix Concatenation1 : Concatenate  Mode=Multidimensional array
  Product{,1..5} (6 alike) : Product  RndMeth=Zero
  Trigonometric Function{,1,6..8,11..12} (7 alike) : Trigonometry  Operator=cos
  Trigonometric Function{2..5,9..10,13} (7 alike) : Trigonometry
  Vector Concatenate : Concatenate  NumInputs=3  Mode=Multidimensional array
  Out1 : Outport
  wires:
  Matrix Concatenation1/1 -> Display/1, Out1/1
  Constant1/1 -> Matrix Concatenation1/2
  Matrix Concatenation/1 -> Matrix Concatenation1/1
  Vector Concatenate/1 -> Matrix Concatenation/2
  A_01/1 -> Matrix Concatenation/1
  Constant5/1 -> Vector Concatenate/3
  Product5/1 -> Vector Concatenate/2
  Product4/1 -> Vector Concatenate/1
  Trigonometric Function13/1 -> Product5/2
  Trigonometric Function12/1 -> Product4/2
  a/1 -> Product5/1, Product4/1
  Trigonometric Function11/1 -> A_01/9
  Trigonometric Function10/1 -> A_01/8
  Constant/1 -> A_01/7
  Gain1/1 -> A_01/6
  Product2/1 -> A_01/5
  Trigonometric Function5/1 -> A_01/4
  Product1/1 -> A_01/3
  Gain/1 -> A_01/2
  Trigonometric Function/1 -> A_01/1
  Product/1 -> Gain/1
  Product3/1 -> Gain1/1
  Trigonometric Function9/1 -> Product3/2
  Trigonometric Function8/1 -> Product3/1
  Trigonometric Function7/1 -> Product2/2
  Trigonometric Function6/1 -> Product2/1
  Trigonometric Function4/1 -> Product1/2
  Trigonometric Function3/1 -> Product1/1
  alpha/1 -> Trigonometric Function11/1, Trigonometric Function10/1, Trigonometric Function9/1, Trigonometric Function7/1, Trigonometric Function4/1, Trigonometric Function1/1
  q/1 -> Trigonometric Function13/1, Trigonometric Function12/1, Trigonometric Function8/1, Trigonometric Function6/1, Trigonometric Function5/1, Trigonometric Function3/1, Trigonometric Function2/1, Trigonometric Function/1
  Trigonometric Function1/1 -> Product/2
  Trigonometric Function2/1 -> Product/1

== system Simulatore_COMAU_UBHAND_IV_manipolazione_interna/Work Space Control/Inverse_kinematic/Inverse_kinematic_comau/R_0index/T_6_index_^-1/Direct_kinematic_wrist1/T_6_8/Subsystem1 ==
  q : Inport
  alpha : Inport  Port=2
  a : Inport  Port=3
  A_01 : aerolibutil/Create 3x3 Matrix
  Constant : Constant  Value=0
  Constant1 : Constant  Value=[0 0 0 1]  VectorParams1D=off
  Constant5 : Constant  Value=d_w(2)
  Display : Display
  Gain : Gain  Gain=-1
  Gain1 : Gain  Gain=-1
  Matrix Concatenation : Concatenate  Mode=Multidimensional array  ConcatenateDimension=2
  Matrix Concatenation1 : Concatenate  Mode=Multidimensional array
  Product{,1..5} (6 alike) : Product  RndMeth=Zero
  Trigonometric Function{,1,6..8,11..12} (7 alike) : Trigonometry  Operator=cos
  Trigonometric Function{2..5,9..10,13} (7 alike) : Trigonometry
  Vector Concatenate : Concatenate  NumInputs=3  Mode=Multidimensional array
  Out1 : Outport
  wires:
  Trigonometric Function2/1 -> Product/1
  Trigonometric Function1/1 -> Product/2
  q/1 -> Trigonometric Function/1, Trigonometric Function2/1, Trigonometric Function3/1, Trigonometric Function5/1, Trigonometric Function6/1, Trigonometric Function8/1, Trigonometric Function12/1, Trigonometric Function13/1
  alpha/1 -> Trigonometric Function1/1, Trigonometric Function4/1, Trigonometric Function7/1, Trigonometric Function9/1, Trigonometric Function10/1, Trigonometric Function11/1
  Trigonometric Function3/1 -> Product1/1
  Trigonometric Function4/1 -> Product1/2
  Trigonometric Function6/1 -> Product2/1
  Trigonometric Function7/1 -> Product2/2
  Trigonometric Function8/1 -> Product3/1
  Trigonometric Function9/1 -> Product3/2
  Product3/1 -> Gain1/1
  Product/1 -> Gain/1
  Trigonometric Function/1 -> A_01/1
  Gain/1 -> A_01/2
  Product1/1 -> A_01/3
  Trigonometric Function5/1 -> A_01/4
  Product2/1 -> A_01/5
  Gain1/1 -> A_01/6
  Constant/1 -> A_01/7
  Trigonometric Function10/1 -> A_01/8
  Trigonometric Function11/1 -> A_01/9
  a/1 -> Product5/1, Product4/1
  Trigonometric Function12/1 -> Product4/2
  Trigonometric Function13/1 -> Product5/2
  Product4/1 -> Vector Concatenate/1
  Product5/1 -> Vector Concatenate/2
  Constant5/1 -> Vector Concatenate/3
  A_01/1 -> Matrix Concatenation/1
  Vector Concatenate/1 -> Matrix Concatenation/2
  Matrix Concatenation/1 -> Matrix Concatenation1/1
  Constant1/1 -> Matrix Concatenation1/2
  Matrix Concatenation1/1 -> Out1/1, Display/1

== system Simulatore_COMAU_UBHAND_IV_manipolazione_interna/Work Space Control/Inverse_kinematic/Inverse_kinematic_comau/R_0index/T_6_index_^-1/Direct_kinematic_wrist1/T_6_8/wrist_reference_ ==
  Angle Conversion : aerolibconvert2/Angle Conversion  IU=deg  OU=rad
  Constant1 : Constant  Value=[0 90 0]  VectorParams1D=off
  Constant2 : Constant  Value=[0 0 0 ]'  VectorParams1D=off
  Constant4 : Constant  Value=[0 0 0 1]  VectorParams1D=off
  Matrix Concatenate : Concatenate  Mode=Multidimensional array  ConcatenateDimension=2
  Matrix Concatenate1 : Concatenate  Mode=Multidimensional array
  Rotation Angles to Direction Cosine Matrix : aerolibtransform2/Rotation Angles to Direction Cosine Matrix  rotationOrder=ZYX
  T_HW : Outport
  wires:
  Matrix Concatenate1/1 -> T_HW/1
  Rotation Angles to Direction Cosine Matrix/1 -> Matrix Concatenate/1
  Constant4/1 -> Matrix Concatenate1/2
  Matrix Concatenate/1 -> Matrix Concatenate1/1
  Angle Conversion/1 -> Rotation Angles to Direction Cosine Matrix/1
  Constant1/1 -> Angle Conversion/1
  Constant2/1 -> Matrix Concatenate/2

== system Simulatore_COMAU_UBHAND_IV_manipolazione_interna/Work Space Control/Inverse_kinematic/Inverse_kinematic_comau/R_0index/T_6_object^-1/Direct_kinematic_wrist1/T_6_8/Subsystem ==
  q : Inport
  alpha : Inport  Port=2
  a : Inport  Port=3
  A_01 : aerolibutil/Create 3x3 Matrix
  Constant : Constant  Value=0
  Constant1 : Constant  Value=[0 0 0 1]  VectorParams1D=off
  Constant5 : Constant  Value=d_w(1)
  Display : Display
  Gain : Gain  Gain=-1
  Gain1 : Gain  Gain=-1
  Matrix Concatenation : Concatenate  Mode=Multidimensional array  ConcatenateDimension=2
  Matrix Concatenation1 : Concatenate  Mode=Multidimensional array
  Product{,1..5} (6 alike) : Product  RndMeth=Zero
  Trigonometric Function{,1,6..8,11..12} (7 alike) : Trigonometry  Operator=cos
  Trigonometric Function{2..5,9..10,13} (7 alike) : Trigonometry
  Vector Concatenate : Concatenate  NumInputs=3  Mode=Multidimensional array
  Out1 : Outport
  wires:
  Trigonometric Function2/1 -> Product/1
  Trigonometric Function1/1 -> Product/2
  q/1 -> Trigonometric Function/1, Trigonometric Function2/1, Trigonometric Function3/1, Trigonometric Function5/1, Trigonometric Function6/1, Trigonometric Function8/1, Trigonometric Function12/1, Trigonometric Function13/1
  alpha/1 -> Trigonometric Function1/1, Trigonometric Function4/1, Trigonometric Function7/1, Trigonometric Function9/1, Trigonometric Function10/1, Trigonometric Function11/1
  Trigonometric Function3/1 -> Product1/1
  Trigonometric Function4/1 -> Product1/2
  Trigonometric Function6/1 -> Product2/1
  Trigonometric Function7/1 -> Product2/2
  Trigonometric Function8/1 -> Product3/1
  Trigonometric Function9/1 -> Product3/2
  Product3/1 -> Gain1/1
  Product/1 -> Gain/1
  Trigonometric Function/1 -> A_01/1
  Gain/1 -> A_01/2
  Product1/1 -> A_01/3
  Trigonometric Function5/1 -> A_01/4
  Product2/1 -> A_01/5
  Gain1/1 -> A_01/6
  Constant/1 -> A_01/7
  Trigonometric Function10/1 -> A_01/8
  Trigonometric Function11/1 -> A_01/9
  a/1 -> Product4/1, Product5/1
  Trigonometric Function12/1 -> Product4/2
  Trigonometric Function13/1 -> Product5/2
  Product4/1 -> Vector Concatenate/1
  Product5/1 -> Vector Concatenate/2
  Constant5/1 -> Vector Concatenate/3
  A_01/1 -> Matrix Concatenation/1
  Vector Concatenate/1 -> Matrix Concatenation/2
  Matrix Concatenation/1 -> Matrix Concatenation1/1
  Constant1/1 -> Matrix Concatenation1/2
  Matrix Concatenation1/1 -> Out1/1, Display/1

== system Simulatore_COMAU_UBHAND_IV_manipolazione_interna/Work Space Control/Inverse_kinematic/Inverse_kinematic_comau/R_0index/T_6_object^-1/Direct_kinematic_wrist1/T_6_8/Subsystem1 ==
  q : Inport
  alpha : Inport  Port=2
  a : Inport  Port=3
  A_01 : aerolibutil/Create 3x3 Matrix
  Constant : Constant  Value=0
  Constant1 : Constant  Value=[0 0 0 1]  VectorParams1D=off
  Constant5 : Constant  Value=d_w(2)
  Display : Display
  Gain : Gain  Gain=-1
  Gain1 : Gain  Gain=-1
  Matrix Concatenation : Concatenate  Mode=Multidimensional array  ConcatenateDimension=2
  Matrix Concatenation1 : Concatenate  Mode=Multidimensional array
  Product{,1..5} (6 alike) : Product  RndMeth=Zero
  Trigonometric Function{,1,6..8,11..12} (7 alike) : Trigonometry  Operator=cos
  Trigonometric Function{2..5,9..10,13} (7 alike) : Trigonometry
  Vector Concatenate : Concatenate  NumInputs=3  Mode=Multidimensional array
  Out1 : Outport
  wires:
  Matrix Concatenation1/1 -> Display/1, Out1/1
  Constant1/1 -> Matrix Concatenation1/2
  Matrix Concatenation/1 -> Matrix Concatenation1/1
  Vector Concatenate/1 -> Matrix Concatenation/2
  A_01/1 -> Matrix Concatenation/1
  Constant5/1 -> Vector Concatenate/3
  Product5/1 -> Vector Concatenate/2
  Product4/1 -> Vector Concatenate/1
  Trigonometric Function13/1 -> Product5/2
  Trigonometric Function12/1 -> Product4/2
  a/1 -> Product4/1, Product5/1
  Trigonometric Function11/1 -> A_01/9
  Trigonometric Function10/1 -> A_01/8
  Constant/1 -> A_01/7
  Gain1/1 -> A_01/6
  Product2/1 -> A_01/5
  Trigonometric Function5/1 -> A_01/4
  Product1/1 -> A_01/3
  Gain/1 -> A_01/2
  Trigonometric Function/1 -> A_01/1
  Product/1 -> Gain/1
  Product3/1 -> Gain1/1
  Trigonometric Function9/1 -> Product3/2
  Trigonometric Function8/1 -> Product3/1
  Trigonometric Function7/1 -> Product2/2
  Trigonometric Function6/1 -> Product2/1
  Trigonometric Function4/1 -> Product1/2
  Trigonometric Function3/1 -> Product1/1
  alpha/1 -> Trigonometric Function11/1, Trigonometric Function10/1, Trigonometric Function9/1, Trigonometric Function7/1, Trigonometric Function4/1, Trigonometric Function1/1
  q/1 -> Trigonometric Function13/1, Trigonometric Function12/1, Trigonometric Function8/1, Trigonometric Function6/1, Trigonometric Function5/1, Trigonometric Function3/1, Trigonometric Function2/1, Trigonometric Function/1
  Trigonometric Function1/1 -> Product/2
  Trigonometric Function2/1 -> Product/1

== system Simulatore_COMAU_UBHAND_IV_manipolazione_interna/Work Space Control/Inverse_kinematic/Inverse_kinematic_comau/R_0index/T_6_object^-1/Direct_kinematic_wrist1/T_6_8/wrist_reference_ ==
  Angle Conversion : aerolibconvert2/Angle Conversion  IU=deg  OU=rad
  Constant1 : Constant  Value=[0 90 0]  VectorParams1D=off
  Constant2 : Constant  Value=[0 0 0 ]'  VectorParams1D=off
  Constant4 : Constant  Value=[0 0 0 1]  VectorParams1D=off
  Matrix Concatenate : Concatenate  Mode=Multidimensional array  ConcatenateDimension=2
  Matrix Concatenate1 : Concatenate  Mode=Multidimensional array
  Rotation Angles to Direction Cosine Matrix : aerolibtransform2/Rotation Angles to Direction Cosine Matrix  rotationOrder=ZYX
  T_HW : Outport
  wires:
  Constant2/1 -> Matrix Concatenate/2
  Constant1/1 -> Angle Conversion/1
  Angle Conversion/1 -> Rotation Angles to Direction Cosine Matrix/1
  Matrix Concatenate/1 -> Matrix Concatenate1/1
  Constant4/1 -> Matrix Concatenate1/2
  Rotation Angles to Direction Cosine Matrix/1 -> Matrix Concatenate/1
  Matrix Concatenate1/1 -> T_HW/1
